simulink model "ClimaManModMgr"  (R2022b)
settings: Solver=FixedStepDiscrete  StartTime=0.0  StopTime=10.0  FixedStep=0.2  RelTol=1e-3
(part 1 of 2)
settings Model: Creator=blue.ge  LastModifiedBy=blue.ge
settings DataIOCC: SaveFormat=StructureWithTime
settings OptimizationCC: DefaultParameterBehavior=Inlined  EnhancedBackFolding=on  PassReuseOutputArgsAs=Individual arguments  ZeroExternalMemoryAtStartup=off  ZeroInternalMemoryAtStartup=off  OptimizeBlockOrder=speed  DifferentSizesBufferReuse=on  OptimizationCustomize=off  EfficientTunableParamExpr=on
settings DebuggingCC: ParameterTunabilityLossMsg=error
settings ModelReferenceCC: ModelReferenceNumInstancesAllowed=Single
settings SFSimCC: MATLABDynamicMemAlloc=off
settings RTWCC: Description=AUTOSAR  SystemTargetFile=autosar.tlc  GenCodeOnly=on  TemplateMakefile=ert_default_tmf
settings CodeAppCC: ForceParamTrailComments=on  IgnoreCustomStorageClasses=off  OperatorAnnotations=on  SimulinkDataObjDesc=on  SFDataObjDesc=on  InsertBlockDesc=on
settings STFCustomTargetCC: TargetLangStandard=C99 (ISO)  MultiwordLength=256  ParMdlRefBuildCompliant=on  CompOptLevelCompliant=on  IncludeMdlTerminateFcn=off  CombineOutputUpdateFcns=on  SuppressErrorStatus=on  ERTCustomFileBanners=on  SupportNonFinite=off  SupportComplex=off  SupportContinuousTime=off  SupportNonInlinedSFcns=off  RemoveResetFunc=on  MATLABClassNameForMDSCustomization=Simulink.SoftwareTarget.ERTCustomization  AutosarCompliant=on  UseToolchainInfoCompliant=on  LookupTableObjectStructAxisOrder=2,1,3,4,...  LUTObjectStructOrderEvenSpacing=Size,Table,Breakpoints  SystemTargetFile=autosar.tlc
settings ERTTargetCC: TargetLangStandard=C99 (ISO)  ConcurrentExecutionCompliant=off  IncludeMdlTerminateFcn=off  SuppressErrorStatus=on  SupportNonFinite=off  SupportComplex=off  ModelStepFunctionPrototypeControlCompliant=off  CPPClassGenCompliant=off  AutosarCompliant=on  LookupTableObjectStructAxisOrder=2,1,3,4,...  LUTObjectStructOrderEvenSpacing=Size,Table,Breakpoints  InlinedParameterPlacement=NonHierarchical  ERTCustomFileTemplate=example_file_process.tlc

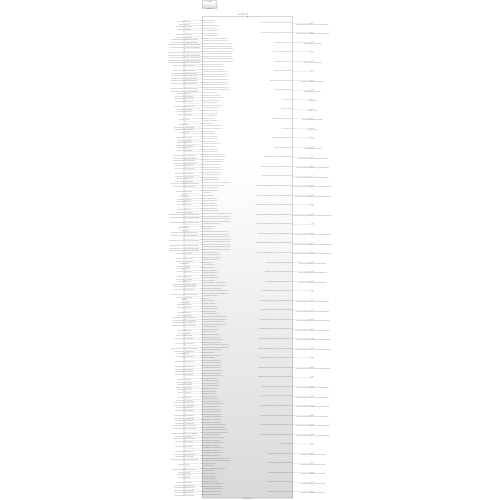
[thumbnail: Simulink's stored preview of the top-level diagram]
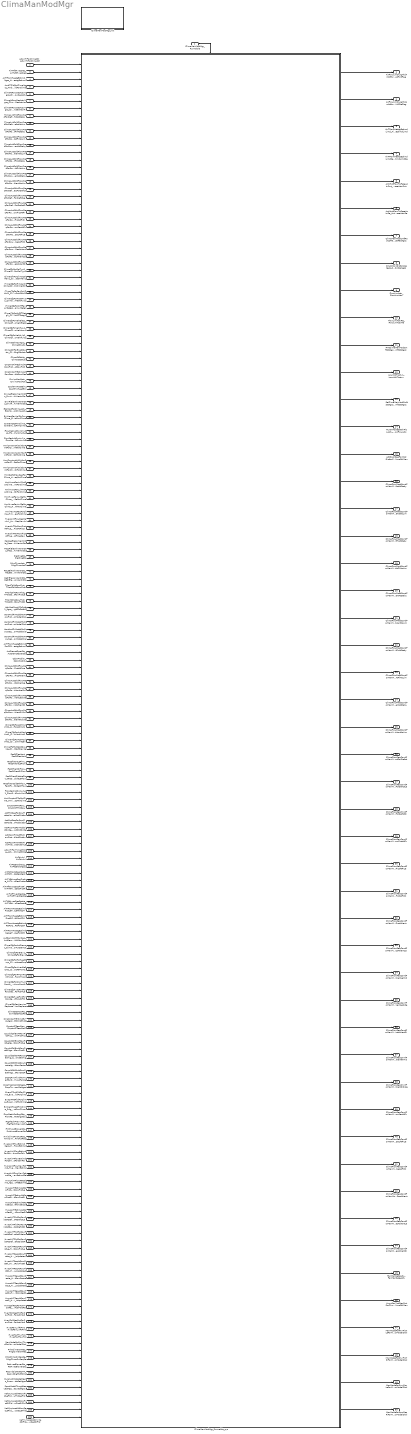
== system ClimaManModMgr ==
  ClimaManModMgr_Runnable : Inport  SampleTime=0.2  OutputFunctionCall=on
  ActvnTiForClimaCtrl_ActvnTiForClimaCtrl : Inport  Port=2  OutDataTypeStr=Ti33  PortDimensions=1
  AirTAftHvacDes_AirTAftHvacDes : Inport  Port=3  OutDataTypeStr=T30  PortDimensions=1
  AirTFromHeatgEstimd_Ngcc_AirTFromHeatgEstimd : Inport  Port=4  OutDataTypeStr=T30  PortDimensions=1
  AmbTFildForClimaNgcc_AmbTFildForClima : Inport  Port=5  OutDataTypeStr=T30  PortDimensions=1
  ClimaAtRowSecActvNgcc_ClimaAtRowSecActv : Inport  Port=6  OutDataTypeStr=Boolean  PortDimensions=1
  ClimaAtRowSecActvNgcc_ClimaAtRowSecActvLe : Inport  Port=7  OutDataTypeStr=Boolean  PortDimensions=1
  ClimaAtRowSecActvNgcc_ClimaAtRowSecActvRi : Inport  Port=8  OutDataTypeStr=Boolean  PortDimensions=1
  ClimaAutCtrldFlowPredForDefrstAtStaby_ClimaAutCtrldFlowPredForDefrstAtStaby : Inport  Port=9  OutDataTypeStr=AirFlow30  PortDimensions=1
  ClimaAutCtrldFlowPredForDefrstNoLim_ClimaAutCtrldFlowPredForDefrstNoLim : Inport  Port=10  OutDataTypeStr=AirFlow30  PortDimensions=1
  ClimaAutCtrldFlowPredForRowFirstAtFlrAtStaby_ClimaAutCtrldFlowPredForRowFirstAtFlrAtStaby : Inport  Port=11  OutDataTypeStr=AirFlow30  PortDimensions=1
  ClimaAutCtrldFlowPredForRowFirstAtFlrNoLim_ClimaAutCtrldFlowPredForRowFirstAtFlrNoLim : Inport  Port=12  OutDataTypeStr=AirFlow30  PortDimensions=1
  ClimaAutCtrldFlowPredForRowFirstAtVentAtStaby_ClimaAutCtrldFlowPredForRowFirstAtVentAtStaby : Inport  Port=13  OutDataTypeStr=AirFlow30  PortDimensions=1
  ClimaAutCtrldFlowPredForRowFirstAtVentNoLim_ClimaAutCtrldFlowPredForRowFirstAtVentNoLim : Inport  Port=14  OutDataTypeStr=AirFlow30  PortDimensions=1
  ClimaAutCtrldFlowPredForRowSecAtFlrAtStaby_ClimaAutCtrldFlowPredForRowSecAtFlrAtStaby : Inport  Port=15  OutDataTypeStr=AirFlow30  PortDimensions=1
  ClimaAutCtrldFlowPredForRowSecAtFlrNoLim_ClimaAutCtrldFlowPredForRowSecAtFlrNoLim : Inport  Port=16  OutDataTypeStr=AirFlow30  PortDimensions=1
  ClimaAutCtrldFlowPredForRowSecAtVentAtStaby_ClimaAutCtrldFlowPredForRowSecAtVentAtStaby : Inport  Port=17  OutDataTypeStr=AirFlow30  PortDimensions=1
  ClimaAutCtrldFlowPredForRowSecAtVentNoLim_ClimaAutCtrldFlowPredForRowSecAtVentNoLim : Inport  Port=18  OutDataTypeStr=AirFlow30  PortDimensions=1
  ClimaAutCtrldFlowReqForDefrst_ClimaAutCtrldFlowReqForDefrst : Inport  Port=19  OutDataTypeStr=AirFlow30  PortDimensions=1
  ClimaAutCtrldFlowReqForDefrst_ClimaAutCtrldFlowReqForDefrstLe : Inport  Port=20  OutDataTypeStr=AirFlow30  PortDimensions=1
  ClimaAutCtrldFlowReqForDefrst_ClimaAutCtrldFlowReqForDefrstRi : Inport  Port=21  OutDataTypeStr=AirFlow30  PortDimensions=1
  ClimaAutCtrldFlowReqForRowFirstAtFlr_ClimaAutCtrldFlowReqForRowFirstAtFlr : Inport  Port=22  OutDataTypeStr=AirFlow30  PortDimensions=1
  ClimaAutCtrldFlowReqForRowFirstAtFlr_ClimaAutCtrldFlowReqForRowFirstAtFlrRi : Inport  Port=23  OutDataTypeStr=AirFlow30  PortDimensions=1
  ClimaAutCtrldFlowReqForRowSecAtFlr_ClimaAutCtrldFlowReqForRowSecAtFlr : Inport  Port=24  OutDataTypeStr=AirFlow30  PortDimensions=1
  ClimaAutCtrldFlowReqForRowSecAtFlr_ClimaAutCtrldFlowReqForRowSecAtFlrLe : Inport  Port=25  OutDataTypeStr=AirFlow30  PortDimensions=1
  ClimaAutCtrldFlowReqForRowSecAtFlr_ClimaAutCtrldFlowReqForRowSecAtFlrRi : Inport  Port=26  OutDataTypeStr=AirFlow30  PortDimensions=1
  ClimaAutCtrldFlowReqForRowSecAtVent_ClimaAutCtrldFlowReqForRowSecAtVent : Inport  Port=27  OutDataTypeStr=AirFlow30  PortDimensions=1
  ClimaAutCtrldFlowReqForRowSecAtVent_ClimaAutCtrldFlowReqForRowSecAtVentLe : Inport  Port=28  OutDataTypeStr=AirFlow30  PortDimensions=1
  ClimaAutCtrldFlowReqForRowSecAtVent_ClimaAutCtrldFlowReqForRowSecAtVentRi : Inport  Port=29  OutDataTypeStr=AirFlow30  PortDimensions=1
  ClimaCfgForCarTyp1_ClimaCfgForCarTyp1 : Inport  Port=30  OutDataTypeStr=Enum: ClimaCfgForCarTyp1  PortDimensions=1
  ClimaCfgForClimaCtrlMan1_ClimaCfgForClimaCtrlMan1 : Inport  Port=31  OutDataTypeStr=Boolean  PortDimensions=1
  ClimaCfgForExtVentnClimaCtrl_ClimaCfgForExtVentnClimaCtrl : Inport  Port=32  OutDataTypeStr=Enum: CarCfgForExtVentnClimaCtrl  PortDimensions=1
  ClimaCfgForFanPotiCtrld1_ClimaCfgForFanPotiCtrld1 : Inport  Port=33  OutDataTypeStr=Boolean  PortDimensions=1
  ClimaCfgForHeatrTyp1_ClimaCfgForHeatrTyp1 : Inport  Port=34  OutDataTypeStr=Enum: ClimaCfgForHeatrTyp1  PortDimensions=1
  ClimaCfgForNrOfFanLvlSetg1_ClimaCfgForNrOfFanLvlSetg1 : Inport  Port=35  OutDataTypeStr=Enum: ClimaCfgForNrOfFanLvlSetg1  PortDimensions=1
  ClimaCfgForNrOfTSetg1_ClimaCfgForNrOfTSetg1 : Inport  Port=36  OutDataTypeStr=Enum: ClimaCfgForNrOfTSetg1  PortDimensions=1
  ClimaCfgForVehElec1_ClimaCfgForVehElec1 : Inport  Port=37  OutDataTypeStr=Boolean  PortDimensions=1
  ClimaCfgForVehHev1_ClimaCfgForVehHev1 : Inport  Port=38  OutDataTypeStr=Boolean  PortDimensions=1
  ClimaCfgForVehHyb1_ClimaCfgForVehHyb1 : Inport  Port=39  OutDataTypeStr=Boolean  PortDimensions=1
  ClimaCtrlActvNgcc_ClimaCtrlActv : Inport  Port=40  OutDataTypeStr=Boolean  PortDimensions=1
  ClimaCtrlForEngStbActv_ClimaCtrlForEngStbActv : Inport  Port=41  OutDataTypeStr=Boolean  PortDimensions=1
  ClimaStStrtUp_ClimaStStrtUp : Inport  Port=42  OutDataTypeStr=Enum: ClimaStStrtUp  PortDimensions=1
  CmptmtAirTEstimdAtRowFirst_CmptmtAirTEstimdAtRowFirst : Inport  Port=43  OutDataTypeStr=T30  PortDimensions=1
  CmptmtAirTEstimdAtRowSec_CmptmtAirTEstimdAtRowSec : Inport  Port=44  OutDataTypeStr=T30  PortDimensions=1
  CnvincModActv_CnvincModActv : Inport  Port=45  OutDataTypeStr=Boolean  PortDimensions=1
  CooltTInHeatElm_CooltTInHeatElm : Inport  Port=46  OutDataTypeStr=T30  PortDimensions=1
  DrvrLeElecAirVentnSts_DrvrLeElecAirVentnSts : Inport  Port=47  OutDataTypeStr=Enum: OnOff1  PortDimensions=1
  DrvrRiElecAirVentnSts_DrvrRiElecAirVentnSts : Inport  Port=48  OutDataTypeStr=Enum: OnOff1  PortDimensions=1
  EcoModForClimaActv_EcoModForClimaActv : Inport  Port=49  OutDataTypeStr=Enum: OnOff1  PortDimensions=1
  ExtVentFanVolStsFrmClima_ExtVentFanVolStsFrmClima : Inport  Port=50  OutDataTypeStr=Enum: Level4  PortDimensions=1
  ExtVentStsFrmClima_ExtVentStsFrmClima : Inport  Port=51  OutDataTypeStr=Enum: OnOff1  PortDimensions=1
  FlowRednAtPwrAllwdLo_FlowRednAtPwrAllwdLo : Inport  Port=52  OutDataTypeStr=Boolean  PortDimensions=1
  FlowRednAtPwrLvlLo_FlowRednAtPwrLvlLo : Inport  Port=53  OutDataTypeStr=Boolean  PortDimensions=1
  HmiCmptmtAirDistbnFrntForClima_HmiCmptmtAirDistbnFrntForClima : Inport  Port=54  OutDataTypeStr=Enum: HmiCmptmtAirDistbnFrnt  PortDimensions=1
  HmiCmptmtAirDistbnFrntForClima_HmiCmptmtAirDistbnFrntRiForClima : Inport  Port=55  OutDataTypeStr=Enum: HmiCmptmtAirDistbnFrnt  PortDimensions=1
  HmiCmptmtAirDistbnFrntForClima_HmiCmptmtAirDistbnReForClima : Inport  Port=56  OutDataTypeStr=Enum: HmiCmptmtAirDistbnFrnt  PortDimensions=1
  HmiCmptmtAirDistbnFrntForClima_HmiCmptmtAirDistbnReRiForClima : Inport  Port=57  OutDataTypeStr=Enum: HmiCmptmtAirDistbnFrnt  PortDimensions=1
  HmiDefrstMaxReqForClima_HmiDefrstMaxReqForClima : Inport  Port=58  OutDataTypeStr=Enum: ActrReq  PortDimensions=1
  HmiHvacFanLvlFrntForClima_HmiHvacFanLvlFrntForClima : Inport  Port=59  OutDataTypeStr=Enum: HmiHvacFanLvl  PortDimensions=1
  HmiHvacFanLvlFrntForClima_HmiHvacFanLvlFrntRiForClima : Inport  Port=60  OutDataTypeStr=Enum: HmiHvacFanLvl  PortDimensions=1
  HmiHvacFanLvlReForClima_HmiHvacFanLvlReForClima : Inport  Port=61  OutDataTypeStr=Enum: HmiHvacFanLvl  PortDimensions=1
  HmiHvacFanLvlReForClima_HmiHvacFanLvlReRiForClima : Inport  Port=62  OutDataTypeStr=Enum: HmiHvacFanLvl  PortDimensions=1
  HmiMaxACReqForClima_HmiMaxACReqForClima : Inport  Port=63  OutDataTypeStr=Enum: OnOff1  PortDimensions=1
  HvacAirMFlowReqMaxAvl_HvacAirMFlowReqMaxAvl : Inport  Port=64  OutDataTypeStr=AirFlow30  PortDimensions=1
  HvacAirTForRowFirstAtFlrLe_HvacAirTForRowFirstAtFlrLe_HvacAirTForRowFirstAtFlrLe : Inport  Port=65  OutDataTypeStr=Bus: HvacAirTForRowFirstAtFlrLe  PortDimensions=1  BusOutputAsStruct=on
  HvacAirTForRowFirstAtFlrLeRaw_HvacAirTForRowFirstAtFlrLeRaw : Inport  Port=66  OutDataTypeStr=Bus: HvacAirTForRowFirstAtFlrLe  PortDimensions=1  BusOutputAsStruct=on
  PassLeElecAirVentnSts_PassLeElecAirVentnSts : Inport  Port=67  OutDataTypeStr=Enum: OnOff1  PortDimensions=1
  PassRiElecAirVentnSts_PassRiElecAirVentnSts : Inport  Port=68  OutDataTypeStr=Enum: OnOff1  PortDimensions=1
  PreClngSts_PreClngSts : Inport  Port=69  OutDataTypeStr=Enum: PreClngSts  PortDimensions=1
  PrkgClimaActv_PrkgClimaActv : Inport  Port=70  OutDataTypeStr=Enum: PrkgClimaActv  PortDimensions=1
  ReLeElecAirVentnSts_ReLeElecAirVentnSts : Inport  Port=71  OutDataTypeStr=Enum: OnOff1  PortDimensions=1
  ReRiElecAirVentnSts_ReRiElecAirVentnSts : Inport  Port=72  OutDataTypeStr=Enum: OnOff1  PortDimensions=1
  TModCtrlAtRowFirst_TModCtrlAtRowFirst : Inport  Port=73  OutDataTypeStr=T31  PortDimensions=1
  TModCtrlAtRowFirst_TModCtrlAtRowFirstLe : Inport  Port=74  OutDataTypeStr=T31  PortDimensions=1
  TModCtrlAtRowFirst_TModCtrlAtRowFirstRi : Inport  Port=75  OutDataTypeStr=T31  PortDimensions=1
  VehModMngtGlbSafe1_Ngcc_VehModMngtGlbSafe1 : Inport  Port=76  OutDataTypeStr=Bus: VehModMngtGlbSafe1  PortDimensions=1  BusOutputAsStruct=on
  VentAndFlrModelForRowFirstLeForModelDial_VentAndFlrModelForRowFirstLeForModelDial : Inport  Port=77  OutDataTypeStr=Enum: HmiCmptmtAirDistbnFrnt  PortDimensions=1
  VentAndFlrModelForRowFirstRiForModelDial_VentAndFlrModelForRowFirstRiForModelDial : Inport  Port=78  OutDataTypeStr=Enum: HmiCmptmtAirDistbnFrnt  PortDimensions=1
  VentAndFlrModelForRowSecLeForModelDial_VentAndFlrModelForRowSecLeForModelDial : Inport  Port=79  OutDataTypeStr=Enum: HmiCmptmtAirDistbnFrnt  PortDimensions=1
  VentAndFlrModelForRowSecRiForModelDial_VentAndFlrModelForRowSecRiForModelDial : Inport  Port=80  OutDataTypeStr=Enum: HmiCmptmtAirDistbnFrnt  PortDimensions=1
  AirTFromHeatgEstimdForCCM_AirTFromHeatgEstimd : Inport  Port=81  OutDataTypeStr=T30  PortDimensions=1
  AutDemstOperSts_AutDemstOperSts : Inport  Port=82  OutDataTypeStr=Enum: OnOff1  PortDimensions=1
  CdsnProblty_CdsnProblty : Inport  Port=83  OutDataTypeStr=Fac30  PortDimensions=1
  ClimaAutCtrldFlowReqForRowFirstAtFlr_ClimaAutCtrldFlowReqForRowFirstAtFlrLe : Inport  Port=84  OutDataTypeStr=AirFlow30  PortDimensions=1
  ClimaAutCtrldFlowReqForRowFirstAtVent_ClimaAutCtrldFlowReqForRowFirstAtVent : Inport  Port=85  OutDataTypeStr=AirFlow30  PortDimensions=1
  ClimaAutCtrldFlowReqForRowFirstAtVent_ClimaAutCtrldFlowReqForRowFirstAtVentLe : Inport  Port=86  OutDataTypeStr=AirFlow30  PortDimensions=1
  ClimaAutCtrldFlowReqForRowFirstAtVent_ClimaAutCtrldFlowReqForRowFirstAtVentLeMid : Inport  Port=87  OutDataTypeStr=AirFlow30  PortDimensions=1
  ClimaAutCtrldFlowReqForRowFirstAtVent_ClimaAutCtrldFlowReqForRowFirstAtVentLeSide : Inport  Port=88  OutDataTypeStr=AirFlow30  PortDimensions=1
  ClimaAutCtrldFlowReqForRowFirstAtVent_ClimaAutCtrldFlowReqForRowFirstAtVentRi : Inport  Port=89  OutDataTypeStr=AirFlow30  PortDimensions=1
  ClimaAutCtrldFlowReqForRowFirstAtVent_ClimaAutCtrldFlowReqForRowFirstAtVentRiMid : Inport  Port=90  OutDataTypeStr=AirFlow30  PortDimensions=1
  ClimaAutCtrldFlowReqForRowFirstAtVent_ClimaAutCtrldFlowReqForRowFirstAtVentRiSide : Inport  Port=91  OutDataTypeStr=AirFlow30  PortDimensions=1
  ClimaCfgForActrModVrnt1_ClimaCfgForActrInd1 : Inport  Port=92  OutDataTypeStr=Enum: ClimaCfgForActrInd1  PortDimensions=1
  ClimaCfgForActrModVrnt1_ClimaCfgForActrModVrnt1 : Inport  Port=93  OutDataTypeStr=Enum: ClimaCfgForActrModVrnt1  PortDimensions=1
  ClimaCfgForActrModVrnt1_ClimaCfgForActrModVrntCen : Inport  Port=94  OutDataTypeStr=Enum: ClimaCfgForActrModVrntCen  PortDimensions=1
  ClimaCfgForStatrtDevice_ClimaCfgForStartDevice : Inport  Port=95  OutDataTypeStr=Enum: StartDevice  PortDimensions=1
  FacOfSecVent_FacOfSecVent : Inport  Port=96  OutDataTypeStr=VCCFac30  PortDimensions=1
  FacOfVentLeFlow_FacOfVentLeFlow : Inport  Port=97  OutDataTypeStr=VCCFac30  PortDimensions=1
  FacOfVentRiFlow_FacOfVentRiFlow : Inport  Port=98  OutDataTypeStr=VCCFac30  PortDimensions=1
  FacOfVentSideLeFlow_FacOfVentSideLeFlow : Inport  Port=99  OutDataTypeStr=VCCFac30  PortDimensions=1
  FacOfVentSideRiFlow_FacOfVentSideRiFlow : Inport  Port=100  OutDataTypeStr=VCCFac30  PortDimensions=1
  FlowRednAtPwrLvlLo1_FlowRednAtPwrLvlLo1 : Inport  Port=101  OutDataTypeStr=Boolean  PortDimensions=1
  HmiCmptmtTSpForClima_HmiCmptmtTSpForClima : Inport  Port=102  OutDataTypeStr=Bus: HmiCmptmtTSp  PortDimensions=1  BusOutputAsStruct=on
  CmptmtTFrntRaw_CmptmtTFrntRaw : Inport  Port=103  OutDataTypeStr=Bus: CmptmtTFrnt  PortDimensions=1  BusOutputAsStruct=on
  ActrFrntReqForRowFirstLeModDistbn_ActrFrntReqForRowFirstLeModDistbn : Inport  Port=104  OutMin=0  OutMax=100  OutDataTypeStr=Perc9  PortDimensions=1
  ActrFrntReqForRowFirstModDistbn_ActrFrntReqForRowFirstModDistbn : Inport  Port=105  OutMin=0  OutMax=100  OutDataTypeStr=Perc9  PortDimensions=1
  ActrPosnActForModDistbnRe_ActrPosnActForModDistbnRe : Inport  Port=106  OutMin=0  OutMax=100  OutDataTypeStr=Perc9  PortDimensions=1
  ActrPosnFrntActForRowFirstModDistbn_ActrPosnFrntActForRowFirstModDistbn : Inport  Port=107  OutMin=0  OutMax=100  OutDataTypeStr=Perc9  PortDimensions=1
  ActrPosnFrntActForRowFirstModDistbnLe_ActrPosnFrntActForRowFirstModDistbnLe : Inport  Port=108  OutMin=0  OutMax=100  OutDataTypeStr=Perc9  PortDimensions=1
  ActvnTiForClimaCtrlRe_ActvnTiForClimaCtrlRe : Inport  Port=109  OutDataTypeStr=Ti33  PortDimensions=1
  AirCdnAvl_AirCdnAvl : Inport  Port=110  OutDataTypeStr=Boolean  PortDimensions=1
  AirPtcEnadNgcc_AirPtcEnadNgcc : Inport  Port=111  OutDataTypeStr=Boolean  PortDimensions=1
  AirPtcModeReqNgcc_AirPtcModeReqNgcc : Inport  Port=112  OutDataTypeStr=Enum: AirPtcModeReq  PortDimensions=1
  AirTAftHvacDesFirstLe_AirTAftHvacDesFirstLe : Inport  Port=113  OutDataTypeStr=T30  PortDimensions=1
  AirTAftHvacDesFirstRi_AirTAftHvacDesFirstRi : Inport  Port=114  OutDataTypeStr=T30  PortDimensions=1
  AirTAftHvacDesRe_AirTAftHvacDesRe : Inport  Port=115  OutDataTypeStr=T30  PortDimensions=1
  AirTAftHvacDesSecLe_AirTAftHvacDesSecLe : Inport  Port=116  OutDataTypeStr=T30  PortDimensions=1
  AirTFromHeatgEstimdFirstLeForCCM_AirTFromHeatgEstimdFirstLeForCCM : Inport  Port=117  OutDataTypeStr=T30  PortDimensions=1
  AirTFromHeatgEstimdFirstRiForCCM_AirTFromHeatgEstimdFirstRiForCCM : Inport  Port=118  OutDataTypeStr=T30  PortDimensions=1
  AirTFromHeatgEstimdReForCCM_AirTFromHeatgEstimdReForCCM : Inport  Port=119  OutDataTypeStr=T30  PortDimensions=1
  AirTFromHeatgEstimdSecLeForCCM_AirTFromHeatgEstimdSecLeForCCM : Inport  Port=120  OutDataTypeStr=T30  PortDimensions=1
  AutDemstWthFanReq_AutDemstWthFanReq : Inport  Port=121  OutDataTypeStr=UInt8  PortDimensions=1
  ClimaCfgForAutDemst_ClimaCfgForAutDemst : Inport  Port=122  OutDataTypeStr=Boolean  PortDimensions=1
  ClimaCfgForErev1_ClimaCfgForErev1 : Inport  Port=123  OutDataTypeStr=Boolean  PortDimensions=1
  ClimaCfgForForHeatPmp_ClimaCfgForForHeatPmp : Inport  Port=124  OutDataTypeStr=Enum: CarCfgForHeatPmpTyp  PortDimensions=1
  ClimaCfgForHvacRePrsnt1_ClimaCfgForHvacRePrsnt1 : Inport  Port=125  OutDataTypeStr=Enum: CarCfgForHvacRe  PortDimensions=1
  ClimaCfgForPTCInFrontHVAC_ClimaCfgForPTCInFrontHVAC : Inport  Port=126  OutDataTypeStr=Enum: FrontHeaterExchanger  PortDimensions=1
  ClimaCfgForSnsrHumPrsnt1_ClimaCfgForSnsrHumPrsnt1 : Inport  Port=127  OutDataTypeStr=Boolean  PortDimensions=1
  ClimaCfgHvacFrntForRowSecForActrTLe_ClimaCfgHvacFrntForRowSecForActrTLe : Inport  Port=128  OutDataTypeStr=Boolean  PortDimensions=1
  ClimaCfgHvacFrntForRowSecForActrTRi_ClimaCfgHvacFrntForRowSecForActrTRi : Inport  Port=129  OutDataTypeStr=Boolean  PortDimensions=1
  ClimaCfgReqVersionReplace_ClimaCfgReqVersionReplace : Inport  Port=130  OutDataTypeStr=UInt8  PortDimensions=1
  ClimaStStrtUpRe_ClimaStStrtUpRe : Inport  Port=131  OutDataTypeStr=Enum: ClimaStStrtUp  PortDimensions=1
  CmptmtAirTEstimdExtd_Ngcc_CmptmtAirTEstimdExtd : Inport  Port=132  OutDataTypeStr=Bus: CmptmtAirTEstimdExtd  PortDimensions=1  BusOutputAsStruct=on
  CmptmtTRearRaw_CmptmtTRearRaw : Inport  Port=133  OutDataTypeStr=Bus: CmptmtTFrnt  PortDimensions=1  BusOutputAsStruct=on
  CoupldCtrlErrAtRowFirstAvg_CoupldCtrlErrAtRowFirstAvg : Inport  Port=134  OutDataTypeStr=T30  PortDimensions=1
  CoupldCtrlErrAtRowFirstLeNgcc_CoupldCtrlErrAtRowFirstLe : Inport  Port=135  OutDataTypeStr=T30  PortDimensions=1
  CoupldCtrlErrAtRowFirstRiNgcc_CoupldCtrlErrAtRowFirstRi : Inport  Port=136  OutDataTypeStr=T30  PortDimensions=1
  CoupldCtrlErrAtRowSecAvg_CoupldCtrlErrAtRowSecAvg : Inport  Port=137  OutDataTypeStr=T30  PortDimensions=1
  CoupldCtrlErrAtRowSecLeNgcc_CoupldCtrlErrAtRowSecLe : Inport  Port=138  OutDataTypeStr=T30  PortDimensions=1
  CoupldCtrlErrAtRowSecRiNgcc_CoupldCtrlErrAtRowSecRi : Inport  Port=139  OutDataTypeStr=T30  PortDimensions=1
  DCDCPwrAllwdForHvacFanRe_DCDCPwrAllwdForHvacFanRe : Inport  Port=140  OutDataTypeStr=UInt8  PortDimensions=1
  DropTopPosnStsNgcc_DropTopPosnStsNgcc : Inport  Port=141  OutDataTypeStr=Enum: PosnSts  PortDimensions=1
  EvaprTFrntFildForClima_EvaprTFrntFildForClima : Inport  Port=142  OutDataTypeStr=T30  PortDimensions=1
  EvaprTReFildForClima_EvaprTReFildForClima : Inport  Port=143  OutDataTypeStr=T30  PortDimensions=1
  ExtVentToastFrmClima_Prkg_ExtVentToastFrmClima : Inport  Port=144  OutDataTypeStr=Enum: ExtVentToastFrmClimaSts  PortDimensions=1
  FlowRednForEngStby_FlowRednForEngStby : Inport  Port=145  OutDataTypeStr=Boolean  PortDimensions=1
  FogFlashPrevnActv_FogFlashPrevnActv : Inport  Port=146  OutDataTypeStr=Boolean  PortDimensions=1
  FrntHvacBlowerSts_FrntHvacBlowerSts : Inport  Port=147  OutDataTypeStr=Enum: HvacFanSts  PortDimensions=1
  HmiClimaFrntAutReq_HmiClimaFrntAutReq : Inport  Port=148  OutDataTypeStr=Enum: OnOff1  PortDimensions=1
  HvacAirMFlowEstimd_Ngcc_HvacAirMFlowEstimd : Inport  Port=149  OutMin=0  OutMax=1000  OutDataTypeStr=MFlow1  PortDimensions=1
  HvacAirMFlowEstimdForCtrlr_HvacAirMFlowEstimdForCtrlr : Inport  Port=150  OutDataTypeStr=AirFlow30  PortDimensions=1
  HvacAirMFlowEstimdForCtrlrRe_HvacAirMFlowEstimdForCtrlrRe : Inport  Port=151  OutDataTypeStr=AirFlow30  PortDimensions=1
  HvacAirMFlowMaxEstimd_HvacAirMFlowMaxEstimd : Inport  Port=152  OutDataTypeStr=AirFlow30  PortDimensions=1
  HvacAirMFlowMaxEstimdRe_HvacAirMFlowMaxEstimdRe : Inport  Port=153  OutDataTypeStr=AirFlow30  PortDimensions=1
  HvacAirMFlowReEstimd_Ngcc_HvacAirMFlowReEstimd : Inport  Port=154  OutMin=0  OutMax=1000  OutDataTypeStr=MFlow1  PortDimensions=1
  HvacAirTEstimdAtRowFirstLe_HvacAirTEstimdAtRowFirstLe : Inport  Port=155  OutDataTypeStr=T30  PortDimensions=1
  HvacAirTEstimdAtRowFirstRi_HvacAirTEstimdAtRowFirstRi : Inport  Port=156  OutDataTypeStr=T30  PortDimensions=1
  HvacAirTEstimdAtRowSecLe_HvacAirTEstimdAtRowSecLe : Inport  Port=157  OutDataTypeStr=T30  PortDimensions=1
  HvacAirTEstimdAtRowSecRi_HvacAirTEstimdAtRowSecRi : Inport  Port=158  OutDataTypeStr=T30  PortDimensions=1
  HvacAirTFildForRowFirstAtDefrstLe_HvacAirTFildForRowFirstAtDefrstLe : Inport  Port=159  OutDataTypeStr=T30  PortDimensions=1
  HvacAirTFildForRowFirstAtDefrstRi_HvacAirTFildForRowFirstAtDefrstRi : Inport  Port=160  OutDataTypeStr=T30  PortDimensions=1
  HvacAirTFildForRowFirstAtDefrstSideLe_HvacAirTFildForRowFirstAtDefrstSideLe : Inport  Port=161  OutDataTypeStr=T30  PortDimensions=1
  HvacAirTFildForRowFirstAtDefrstSideRi_HvacAirTFildForRowFirstAtDefrstSideRi : Inport  Port=162  OutDataTypeStr=T30  PortDimensions=1
  HvacAirTReqAtRowFirstLe_HvacAirTReqAtRowFirstLe : Inport  Port=163  OutDataTypeStr=T30  PortDimensions=1
  HvacAirTReqAtRowFirstLe_HvacAirTReqAtRowFirstLe_IsUpdated : Inport  Port=164  OutDataTypeStr=boolean  PortDimensions=1
  HvacAirTReqAtRowFirstRi_HvacAirTReqAtRowFirstRi : Inport  Port=165  OutDataTypeStr=T30  PortDimensions=1
  HvacAirTReqAtRowFirstRi_HvacAirTReqAtRowFirstRi_IsUpdated : Inport  Port=166  OutDataTypeStr=boolean  PortDimensions=1
  HvacAirTReqAtRowSecLe_HvacAirTReqAtRowSecLe : Inport  Port=167  OutDataTypeStr=T30  PortDimensions=1
  HvacAirTReqAtRowSecLe_HvacAirTReqAtRowSecLe_IsUpdated : Inport  Port=168  OutDataTypeStr=boolean  PortDimensions=1
  HvacAirTReqAtRowSecRi_HvacAirTReqAtRowSecRi : Inport  Port=169  OutDataTypeStr=T30  PortDimensions=1
  HvacAirTReqAtRowSecRi_HvacAirTReqAtRowSecRi_IsUpdated : Inport  Port=170  OutDataTypeStr=boolean  PortDimensions=1
  HvacCtrlReqForActrModRe_HvacCtrlReqForActrModRe : Inport  Port=171  OutDataTypeStr=Perc30  PortDimensions=1
  HvacCtrlrReqFrntForRowFirstForActrMod_HvacCtrlrReqFrntForRowFirstForActrMod : Inport  Port=172  OutDataTypeStr=Perc30  PortDimensions=1
  HvacCtrlrReqFrntForRowFirstLeForActrMod_HvacCtrlrReqFrntForRowFirstLeForActrMod : Inport  Port=173  OutDataTypeStr=Perc30  PortDimensions=1
  HvacFanLvlFbFrnt_HvacFanLvlFbFrnt : Inport  Port=174  OutDataTypeStr=Enum: HmiHvacFanLvl  PortDimensions=1
  HvacTarFlowTot_HvacTarFlowTot : Inport  Port=175  OutDataTypeStr=AirFlow30  PortDimensions=1
  ManModeForRowThrdForModelDial_ManModeForRowThrdForModelDial : Inport  Port=176  OutDataTypeStr=Enum: HmiCmptmtAirDistbnFrnt  PortDimensions=1
  PrkgClimaOnReq_PrkgClimaOnReq : Inport  Port=177  OutDataTypeStr=Boolean  PortDimensions=1
  PrkgClimaOnReqRe_PrkgClimaOnReqRe : Inport  Port=178  OutDataTypeStr=Enum: OnOff1  PortDimensions=1
  ReHvacBlowerSts_ReHvacBlowerSts : Inport  Port=179  OutDataTypeStr=Enum: HvacFanSts  PortDimensions=1
  ResrvdSigForECM4_ResrvdSigForECM4 : Inport  Port=180  OutDataTypeStr=BattSnsrChksTbl  PortDimensions=1
  RuranACModeSetNgcc_RuranACModeSetNgcc : Inport  Port=181  OutDataTypeStr=Enum: RuranACModeSet  PortDimensions=1
  SportModeThmgtRequestNgcc_SportModeThmgtRequestNgcc : Inport  Port=182  OutDataTypeStr=Enum: ThmgtLevel  PortDimensions=1
  VehSunLoadAtRowFirstLeFild_VehSunLoadAtRowFirstLeFild : Inport  Port=183  OutDataTypeStr=SunLoad30  PortDimensions=1
  VehSunLoadAtRowFirstRiFild_VehSunLoadAtRowFirstRiFild : Inport  Port=184  OutDataTypeStr=SunLoad30  PortDimensions=1
  VehSunLoadAtRowSecLeFild_VehSunLoadAtRowSecLeFild : Inport  Port=185  OutDataTypeStr=SunLoad30  PortDimensions=1
  VehSunLoadAtRowSecRiFild_VehSunLoadAtRowSecRiFild : Inport  Port=186  OutDataTypeStr=SunLoad30  PortDimensions=1
  ClimaManModMgr_Init : Subsystem
  ClimaManModMgr_Runnable_sys : Subsystem
  AirFlowLimDurgMidAmbtForNVHForHVACFrntFlag_AirFlowLimDurgMidAmbtForNVHForHVACFrntFlag : Outport  OutDataTypeStr=Boolean  PortDimensions=1
  AirFlowLimDurgMidAmbtForNVHForHVACReFlag_AirFlowLimDurgMidAmbtForNVHForHVACReFlag : Outport  Port=2  OutDataTypeStr=Boolean  PortDimensions=1
  AirTFromHeatgEstimdLimd_AirTFromHeatgEstimdLimd : Outport  Port=3  OutDataTypeStr=VCCT30  PortDimensions=1
  AirTFromHeatgEstimdLimdRe_AirTFromHeatgEstimdLimdRe : Outport  Port=4  OutDataTypeStr=VCCT30  PortDimensions=1
  AntiHotFlowToFaceActvFrnt_AntiHotFlowToFaceActvFrnt : Outport  Port=5  OutDataTypeStr=Boolean  PortDimensions=1
  AntiHotFlowToFaceActvRe_AntiHotFlowToFaceActvRe : Outport  Port=6  OutDataTypeStr=Boolean  PortDimensions=1
  ClimaManModCmftPopUpReqNgcc_ClimaManModCmftPopUpReqNgcc : Outport  Port=7  OutDataTypeStr=Enum: PopUpReq  PortDimensions=1
  CmptmtAirDistbnRspNgcc_CmptmtAirDistbnRspNgcc : Outport  Port=8  OutDataTypeStr=Bus: CmptmtAirDistbnRsp  PortDimensions=1  BusOutputAsStruct=on
  FlowLimActv_FlowLimActv : Outport  Port=9  OutDataTypeStr=Boolean  PortDimensions=1
  FlowLimActvRe_FlowLimActvRe : Outport  Port=10  OutDataTypeStr=Boolean  PortDimensions=1
  FrntClimaManModCmftStsNgcc_FrntClimaManModCmftStsNgcc : Outport  Port=11  OutDataTypeStr=Enum: ClimaManModCmftSts  PortDimensions=1
  LowrWtrTActvn_LowrWtrTActvn : Outport  Port=12  OutDataTypeStr=Boolean  PortDimensions=1
  ReClimaManModCmftStsNgcc_ReClimaManModCmftStsNgcc : Outport  Port=13  OutDataTypeStr=Enum: ClimaManModCmftSts  PortDimensions=1
  RuranModeSetAirFlowActv_RuranModeSetAirFlowActv : Outport  Port=14  OutDataTypeStr=Boolean  PortDimensions=1
  ActrPosnReqForModDistbnForClimaCtrlMan_ActrPosnReqForModDistbnForClimaCtrlMan : Outport  Port=15  OutDataTypeStr=Perc30  PortDimensions=1
  ClimaFlowPredCorrdForManModForDefrstAtStaby_ClimaFlowPredCorrdForManModForDefrstAtStaby : Outport  Port=16  OutDataTypeStr=AirFlow30  PortDimensions=1
  ClimaFlowPredCorrdForManModForDefrstNoLim_ClimaFlowPredCorrdForManModForDefrstNoLim : Outport  Port=17  OutDataTypeStr=AirFlow30  PortDimensions=1
  ClimaFlowPredCorrdForManModForRowFirstAtFlrAtStaby_ClimaFlowPredCorrdForManModForRowFirstAtFlrAtStaby : Outport  Port=18  OutDataTypeStr=AirFlow30  PortDimensions=1
  ClimaFlowPredCorrdForManModForRowFirstAtFlrNoLim_ClimaFlowPredCorrdForManModForRowFirstAtFlrNoLim : Outport  Port=19  OutDataTypeStr=AirFlow30  PortDimensions=1
  ClimaFlowPredCorrdForManModForRowFirstAtVentAtStaby_ClimaFlowPredCorrdForManModForRowFirstAtVentAtStaby : Outport  Port=20  OutDataTypeStr=AirFlow30  PortDimensions=1
  ClimaFlowPredCorrdForManModForRowFirstAtVentNoLim_ClimaFlowPredCorrdForManModForRowFirstAtVentNoLim : Outport  Port=21  OutDataTypeStr=AirFlow30  PortDimensions=1
  ClimaFlowPredCorrdForManModForRowSecAtFlrAtStaby_ClimaFlowPredCorrdForManModForRowSecAtFlrAtStaby : Outport  Port=22  OutDataTypeStr=AirFlow30  PortDimensions=1
  ClimaFlowPredCorrdForManModForRowSecAtFlrNoLim_ClimaFlowPredCorrdForManModForRowSecAtFlrNoLim : Outport  Port=23  OutDataTypeStr=AirFlow30  PortDimensions=1
  ClimaFlowPredCorrdForManModForRowSecAtVentAtStaby_ClimaFlowPredCorrdForManModForRowSecAtVentAtStaby : Outport  Port=24  OutDataTypeStr=AirFlow30  PortDimensions=1
  ClimaFlowPredCorrdForManModForRowSecAtVentNoLim_ClimaFlowPredCorrdForManModForRowSecAtVentNoLim : Outport  Port=25  OutDataTypeStr=AirFlow30  PortDimensions=1
  ClimaFlowReqCorrdForManModForDefrst_ClimaFlowReqCorrdForManModForDefrst : Outport  Port=26  OutDataTypeStr=AirFlow30  PortDimensions=1
  ClimaFlowReqCorrdForManModForDefrst_ClimaFlowReqCorrdForManModForDefrstLe : Outport  Port=27  OutDataTypeStr=AirFlow30  PortDimensions=1
  ClimaFlowReqCorrdForManModForDefrst_ClimaFlowReqCorrdForManModForDefrstRi : Outport  Port=28  OutDataTypeStr=AirFlow30  PortDimensions=1
  ClimaFlowReqCorrdForManModForRowFirstAtFlr_ClimaFlowReqCorrdForManModForRowFirstAtFlr : Outport  Port=29  OutDataTypeStr=AirFlow30  PortDimensions=1
  ClimaFlowReqCorrdForManModForRowFirstAtFlr_ClimaFlowReqCorrdForManModForRowFirstAtFlrLe : Outport  Port=30  OutDataTypeStr=AirFlow30  PortDimensions=1
  ClimaFlowReqCorrdForManModForRowFirstAtFlr_ClimaFlowReqCorrdForManModForRowFirstAtFlrRi : Outport  Port=31  OutDataTypeStr=AirFlow30  PortDimensions=1
  ClimaFlowReqCorrdForManModForRowFirstAtVent_ClimaFlowReqCorrdForManModForRowFirstAtVent : Outport  Port=32  OutDataTypeStr=AirFlow30  PortDimensions=1
  ClimaFlowReqCorrdForManModForRowFirstAtVent_ClimaFlowReqCorrdForManModForRowFirstAtVentLe : Outport  Port=33  OutDataTypeStr=AirFlow30  PortDimensions=1
  ClimaFlowReqCorrdForManModForRowFirstAtVent_ClimaFlowReqCorrdForManModForRowFirstAtVentLeMid : Outport  Port=34  OutDataTypeStr=AirFlow30  PortDimensions=1
  ClimaFlowReqCorrdForManModForRowFirstAtVent_ClimaFlowReqCorrdForManModForRowFirstAtVentLeSide : Outport  Port=35  OutDataTypeStr=AirFlow30  PortDimensions=1
  ClimaFlowReqCorrdForManModForRowFirstAtVent_ClimaFlowReqCorrdForManModForRowFirstAtVentRi : Outport  Port=36  OutDataTypeStr=AirFlow30  PortDimensions=1
  ClimaFlowReqCorrdForManModForRowFirstAtVent_ClimaFlowReqCorrdForManModForRowFirstAtVentRiMid : Outport  Port=37  OutDataTypeStr=AirFlow30  PortDimensions=1
  ClimaFlowReqCorrdForManModForRowFirstAtVent_ClimaFlowReqCorrdForManModForRowFirstAtVentRiSide : Outport  Port=38  OutDataTypeStr=AirFlow30  PortDimensions=1
  ClimaFlowReqCorrdForManModForRowSecAtFlr_ClimaFlowReqCorrdForManModForRowSecAtFlr : Outport  Port=39  OutDataTypeStr=AirFlow30  PortDimensions=1
  ClimaFlowReqCorrdForManModForRowSecAtFlr_ClimaFlowReqCorrdForManModForRowSecAtFlrLe : Outport  Port=40  OutDataTypeStr=AirFlow30  PortDimensions=1
  ClimaFlowReqCorrdForManModForRowSecAtFlr_ClimaFlowReqCorrdForManModForRowSecAtFlrRi : Outport  Port=41  OutDataTypeStr=AirFlow30  PortDimensions=1
  ClimaFlowReqCorrdForManModForRowSecAtVent_ClimaFlowReqCorrdForManModForRowSecAtVent : Outport  Port=42  OutDataTypeStr=AirFlow30  PortDimensions=1
  ClimaFlowReqCorrdForManModForRowSecAtVent_ClimaFlowReqCorrdForManModForRowSecAtVentLe : Outport  Port=43  OutDataTypeStr=AirFlow30  PortDimensions=1
  ClimaFlowReqCorrdForManModForRowSecAtVent_ClimaFlowReqCorrdForManModForRowSecAtVentRi : Outport  Port=44  OutDataTypeStr=AirFlow30  PortDimensions=1
  FanManSetgActv_FanManSetgActv : Outport  Port=45  OutDataTypeStr=Boolean  PortDimensions=1
  HvacFanSpdReqFrntForClimaCtrlMan_HvacFanSpdReqFrntForClimaCtrlMan : Outport  Port=46  OutDataTypeStr=FanSpd1  PortDimensions=1
  ManModeForRowFirstLeForModelDial_ManModeForRowFirstLeForModelDial : Outport  Port=47  OutDataTypeStr=Enum: HmiCmptmtAirDistbnFrnt  PortDimensions=1
  ManModeForRowFirstRiForModelDial_ManModeForRowFirstRiForModelDial : Outport  Port=48  OutDataTypeStr=Enum: HmiCmptmtAirDistbnFrnt  PortDimensions=1
  ManModeForRowSecLeForModelDial_ManModeForRowSecLeForModelDial : Outport  Port=49  OutDataTypeStr=Enum: HmiCmptmtAirDistbnFrnt  PortDimensions=1
  ManModeForRowSecRiForModelDial_ManModeForRowSecRiForModelDial : Outport  Port=50  OutDataTypeStr=Enum: HmiCmptmtAirDistbnFrnt  PortDimensions=1
  wires:
  ActvnTiForClimaCtrl_ActvnTiForClimaCtrl/1 -> ClimaManModMgr_Runnable_sys/1(ActvnTiForClimaCtrl_ActvnTiForClimaCtrl_read)
  AirTAftHvacDes_AirTAftHvacDes/1 -> ClimaManModMgr_Runnable_sys/2(AirTAftHvacDes_AirTAftHvacDes_read)
  AirTFromHeatgEstimd_Ngcc_AirTFromHeatgEstimd/1 -> ClimaManModMgr_Runnable_sys/3(AirTFromHeatgEstimd_Ngcc_AirTFromHeatgEstimd_read)
  AmbTFildForClimaNgcc_AmbTFildForClima/1 -> ClimaManModMgr_Runnable_sys/4(AmbTFildForClimaNgcc_AmbTFildForClima_read)
  ClimaAtRowSecActvNgcc_ClimaAtRowSecActv/1 -> ClimaManModMgr_Runnable_sys/5(ClimaAtRowSecActvNgcc_ClimaAtRowSecActv_read)
  ClimaAtRowSecActvNgcc_ClimaAtRowSecActvLe/1 -> ClimaManModMgr_Runnable_sys/6(ClimaAtRowSecActvNgcc_ClimaAtRowSecActvLe_read)
  ClimaAtRowSecActvNgcc_ClimaAtRowSecActvRi/1 -> ClimaManModMgr_Runnable_sys/7(ClimaAtRowSecActvNgcc_ClimaAtRowSecActvRi_read)
  ClimaAutCtrldFlowPredForDefrstAtStaby_ClimaAutCtrldFlowPredForDefrstAtStaby/1 -> ClimaManModMgr_Runnable_sys/8(ClimaAutCtrldFlowPredForDefrstAtStaby_ClimaAutCtrldFlowPredForDefrstAtStaby_read)
  ClimaAutCtrldFlowPredForDefrstNoLim_ClimaAutCtrldFlowPredForDefrstNoLim/1 -> ClimaManModMgr_Runnable_sys/9(ClimaAutCtrldFlowPredForDefrstNoLim_ClimaAutCtrldFlowPredForDefrstNoLim_read)
  ClimaAutCtrldFlowPredForRowFirstAtFlrAtStaby_ClimaAutCtrldFlowPredForRowFirstAtFlrAtStaby/1 -> ClimaManModMgr_Runnable_sys/10(ClimaAutCtrldFlowPredForRowFirstAtFlrAtStaby_ClimaAutCtrldFlowPredForRowFirstAtFlrAtStaby_read)
  ClimaAutCtrldFlowPredForRowFirstAtFlrNoLim_ClimaAutCtrldFlowPredForRowFirstAtFlrNoLim/1 -> ClimaManModMgr_Runnable_sys/11(ClimaAutCtrldFlowPredForRowFirstAtFlrNoLim_ClimaAutCtrldFlowPredForRowFirstAtFlrNoLim_read)
  ClimaAutCtrldFlowPredForRowFirstAtVentAtStaby_ClimaAutCtrldFlowPredForRowFirstAtVentAtStaby/1 -> ClimaManModMgr_Runnable_sys/12(ClimaAutCtrldFlowPredForRowFirstAtVentAtStaby_ClimaAutCtrldFlowPredForRowFirstAtVentAtStaby_read)
  ClimaAutCtrldFlowPredForRowFirstAtVentNoLim_ClimaAutCtrldFlowPredForRowFirstAtVentNoLim/1 -> ClimaManModMgr_Runnable_sys/13(ClimaAutCtrldFlowPredForRowFirstAtVentNoLim_ClimaAutCtrldFlowPredForRowFirstAtVentNoLim_read)